simulink model "fft_16k_core"  (Simulink 7.4)
settings: Solver=ode45  StartTime=0.0  StopTime=10.0  RelTol=1e-3
libraries: xbsIndex_r4 x2252, casper_library_misc x100, casper_library_delays x17, casper_library_ffts_twiddle x1
(part 12 of 41)

== system fft_16k_core/fft_wideband_real/fft_direct/butterfly2_0/twiddle_general_4mult ==
  a : Inport
  b : Inport  Port=2
  sync : Inport  Port=3
  AddSub : xbsIndex_r4/AddSub  mode=Subtraction  use_carryin=off  use_carryout=off  en=off  latency=add_latency  precision=Full  arith_type=Unsigned  n_bits=16  bin_pt=14  quantization=Truncate  overflow=Wrap  dbl_ovrd=off  use_behavioral_HDL=on  hw_selection=Fabric  pipelined=off  block_type=addsub
  AddSub1 : xbsIndex_r4/AddSub  mode=Addition  use_carryin=off  use_carryout=off  en=off  latency=add_latency  precision=Full  arith_type=Unsigned  n_bits=16  bin_pt=14  quantization=Truncate  overflow=Wrap  dbl_ovrd=off  use_behavioral_HDL=on  hw_selection=Fabric  pipelined=off  block_type=addsub
  c_to_ri1 : casper_library_misc/c_to_ri  SystemSampleTime=-1  n_bits=input_bit_width  bin_pt=input_bit_width-1
  c_to_ri2 : casper_library_misc/c_to_ri  SystemSampleTime=-1  n_bits=coeff_bit_width  bin_pt=coeff_bit_width-2
      settings: AttributesFormatString=18_16 r/i
  c_to_ri3 : casper_library_misc/c_to_ri  SystemSampleTime=-1  n_bits=input_bit_width  bin_pt=input_bit_width-1
  coeff_gen : Subsystem  AncestorBlock=casper_library_ffts_twiddle_coeff_gen/coeff_gen  mask(coeff_gen){Coeffs=[1+0i       0.99999992646571789212117664646939-0.0003834951875713955632071772150482i       0.99999970586288222662574298738036-0.0007669903187427044854995727973801i       0.99999933819152553304832053981954-0.0011504853371138484743191332526635i       0.99999882345170187925020854891045-0.0015339801862847655001403923691328i       0.99999816164348698244168645032914-0.0019174748098554190121889373443764i        0.99999735276697820918201387030422-0.002300969151425804985755529941116i        0.9999963968222943533348257005855-0.0026844631545959616800156588567461i       0.99999529380957619117964441102231-0.0030679567629659761432425746363606i        0.99999404372898581527806527446955-0.003451449920135993970626842042293i       0.99999264658070718958526867936598-0.0038349425697062275442128509439499i       0.99999110236494559433850781715591-0.0042184346552769629717927202250394i       0.99998941108192840321322591989883-0.0046019261204485704952471536444136i       0.99998757273190408412233409762848-0.0049854169088215105620776057548937i       0.99998558731514319841693350099376-0.0053689069639963424990236617873052i       0.99998345483193773475250054616481-0.0057523962295737357877656314997239i       0.99998117528260110908888691483298-0.0061358846491544752690949771078976i        0.9999787486674688308241343293048-0.0065193721663394680818082171924743i       0.99997617498689761461605485237669-0.0069028587247297558057712585366517i        0.9999734542412659354937431999133-0.0072863442679265214008133000334055i        0.99997058643097413987987920336309-0.007669828739531097012982474581122i       0.99996757155644377945691303466447-0.0080533120831449717808014909792291i       0.99996440961811827730087998133968-0.0084367942423697987741615378354254i       0.99996110061646281685909798397915-0.0088202751608074114741953053453472i       0.99995764455196389786095778617891-0.0092037547820598194364682953505508i       0.99995404142512978040713278460316-0.0095872330497292247708518431181801i         0.9999502912364904849695790289843-0.009970709907418030815140497225002i        0.99994639398659745932462783457595-0.010354185298728842135052019557406i        0.99994234967602391161989316969994-0.010737659167264490545079524963512i        0.99993815830536458832966673071496-0.011121131456628021230703673438711i        0.99993381987523599629952286704793-0.011504602110422713565074381847353i        0.99992933438627606967941119364696-0.011888071072252091517351679783587i        0.99992470183914450299056397852837-0.012271538285719925387429185548172i        0.99991992223452275112549614277668-0.012655003694430242214274962009313i        0.99991499557311347423649294796633-0.013038467241987334449548896486704i        0.99990992185564153693633215880254-0.013421928871995765161773128681943i        0.99990470108285289807525941796484-0.013805388528060390587737238377031i        0.99989933325551538789710548371659-0.014188846153786344519986961643099i        0.99989381837441848599468130487367-0.014572301692779064327676330492523i         0.99988815644037332130977802080452-0.01495575508864429616073810080934i        0.99988234745421256111086449891445-0.015339206284988100154054180279672i        0.99987639141679041099308733464568-0.015722655225416857366349532298955i        0.99987028832898294794517823902424-0.016106101853537287127426935740004i         0.99986403819168767626024418859743-0.01648954611295643662982612909218i        0.99985764100582386060267481298069-0.016872987947281710419122902067102i        0.99985109677233219294123500731075-0.017256427300120877332822999505879i        0.99984440549217523663827478230814-0.017639864115082053153127361611041i          0.9998375671663370933828218767303-0.01802329833577374570974249934352i        0.99983058179582340319058175737155-0.018406729905804820185410974886508i        0.99982344938166156644854254409438-0.018790158768784554627062632903289i        0.99981616992490041084806762228254-0.019173584868322622598579840769162i ...(+319546 chars)], coeff_bit_width=coeff_bit_width, StepPeriod=0, bram_latency=bram_latency, coeffs_bram=on}
      mask prompts: Coeffs "Coefficients: (0 to 2^(FFTSize-1)-1)"; coeff_bit_width "Coefficient Bit Width:"; StepPeriod "Step Period: (2^?)"; bram_latency "Latency:"; coeffs_bram "Store coefficients in BRAM"
      mask help: Generates a sequence of complex coefficients
      mask init:
      | coeff_gen_init( gcb, ...
      |     'Coeffs', Coeffs, ...
      |     'coeff_bit_width', coeff_bit_width, ...
      |     'StepPeriod', StepPeriod, ...
      |     'bram_latency', bram_latency, ...
      |     'coeffs_bram', coeffs_bram);
      settings: AttributesFormatString=4096 @ (18,16)
      mask CallbackString:
      | Coeffs = eval(get_param(gcb, 'Coeffs'));
      | if( length(Coeffs) == 1 ),
      |     set_param(gcb, 'MaskVisibilities',{'on','on','off','off','off'});
      | else
      |     set_param(gcb, 'MaskVisibilities',{'on','on','on','on','on'});
      | end||||
  convert0 : xbsIndex_r4/Convert  arith_type="Signed  (2's comp)"  n_bits=input_bit_width+4  bin_pt=input_bit_width+1  quantization="Round  (unbiased: +/- Inf)"  overflow=Wrap  latency=conv_latency  dbl_ovrd=off  pipeline=on  block_type=convert
      settings: block_version=11.4
  convert1 : xbsIndex_r4/Convert  arith_type="Signed  (2's comp)"  n_bits=input_bit_width+4  bin_pt=input_bit_width+1  quantization="Round  (unbiased: +/- Inf)"  overflow=Wrap  latency=conv_latency  dbl_ovrd=off  pipeline=on  block_type=convert
      settings: block_version=11.4
  delay0 : xbsIndex_r4/Delay  en=off  latency=mult_latency + add_latency + bram_latency + conv_latency  dbl_ovrd=off  reg_retiming=off  block_type=delay
      settings: block_version=11.4
  delay1 : xbsIndex_r4/Delay  en=off  latency=bram_latency  dbl_ovrd=off  reg_retiming=on  block_type=delay
      settings: block_version=11.4
  delay2 : xbsIndex_r4/Delay  en=off  latency=mult_latency + add_latency + bram_latency + conv_latency  dbl_ovrd=off  reg_retiming=off  block_type=delay
      settings: block_version=11.4
  mult{,1..3} (4 alike) : xbsIndex_r4/Mult  precision=Full  arith_type="Signed  (2's comp)"  n_bits=16  bin_pt=14  quantization=Truncate  overflow=Wrap  en=off  latency=mult_latency  dbl_ovrd=off  use_behavioral_HDL=on  opt=Speed  use_embedded=off  optimum_pipeline=off  pipeline=on  use_rpm=on  placement_style=Triangular  block_type=mult
      settings: block_version=11.4
  a_re : Outport
  a_im : Outport  Port=2
  bw_re : Outport  Port=3
  bw_im : Outport  Port=4
  sync_out : Outport  Port=5
  wires:
  a/1 -> delay0/1
  delay0/1 -> c_to_ri1/1
  c_to_ri1/1 -> a_re/1
  c_to_ri1/2 -> a_im/1
  sync/1 -> delay2/1, coeff_gen/1(rst)
  delay2/1 -> sync_out/1
  coeff_gen/1(w) -> c_to_ri2/1
  b/1 -> delay1/1
  delay1/1 -> c_to_ri3/1
  c_to_ri3/1 -> mult/1, mult3/1
  c_to_ri2/1 -> mult/2, mult1/2
  c_to_ri3/2 -> mult1/1, mult2/1
  c_to_ri2/2 -> mult2/2, mult3/2
  mult/1 -> AddSub/1
  mult1/1 -> AddSub1/1
  mult2/1 -> AddSub/2
  mult3/1 -> AddSub1/2
  AddSub/1 -> convert0/1
  convert0/1 -> bw_re/1
  AddSub1/1 -> convert1/1
  convert1/1 -> bw_im/1
